simulink model "TosAeb"  (R2021a)
settings: Solver=FixedStepDiscrete  StartTime=0.0  StopTime=inf  FixedStep=0.01  RelTol=1e-3
undefined symbols (not defined in the file or in its folder): ACCModSts, AccSts1, AEB_ObjectMovingOrientation, AEB_ObjectMovingState, CN_BTCActive, CN_CurrentGearR, CN_CurrentGearR_DHT, CN_PTCActive, CN_VDCActive, CN_VSC_EspOff, CN_VSC_FCW_AEB_Error, CN_VSC_FCW_AEB_NoDispay, CN_VSC_FCW_AEB_PerfmDgdtn, CN_VSC_FCW_AEB_TempUnAvlbl, CN_VSC_VehStandstill, CNaccelAvoidMax, CNaccelAvoidMin, CNbrakePedalPress, CNcomfortStrLeftAccelMax, CNcomfortStrLeftAccelMin, CNcomfortStrRightAccelMax, CNcomfortStrRightAccelMin, CNDrvDoorOpen, CNDrvModeEco, CNDrvModeNorm, CNDrvModeOther, CNDrvModeSport, CNDrvSBRreminder, CNNull, CNobjInvalidID, CNPassDoorOpen, CNPassSBRreminder, CNPEBSAccelNegInf, CNPEBSAccelNull, CNPEBSTimeInf, CNPEBSTimeNull, CNSTOPMAX, CNSTOPNULL, CNsubjWidth, CNSwtOFF, CNSwtON, CNTTCMAX, CNTTCNULL, CNVehConfigAEBCycNone, CNVehConfigAEBNone, CNVehConfigAEBPedNone, CNVehConfigFCWNone, CNVehConfigJANone, CollisionSeverity, FALSE, FCWSnvty, JACycObjCalcInfoDefaultParams, JAPedObjCalcInfoDefaultParams, JAVehObjCalcInfoDefaultParams, latCycObjCalcInfoDefaultParams, latPedObjCalcInfoDefaultParams, longObjCalcInfoDefaultParams, ModSts, movingStatus, ObjectType ... (+1112)
libraries: simulink x3031, AEBSFunctionLib x50, Ta_Library x33, AEBSStateMachineLib x23, Mylibrary x12, simulink_extras x7, ObjSelect_lib x3, dspstat3 x2
(part 68 of 102)

== system TosAeb/FcwAebJa_Step_sys/ALG_AEBSystem/ALG_AEBController/AEB_FuncCondCalcApp/CycFunctionCondCalc/cycAEBpartSuppAborCond/cycAEBpartSuppCondCalc/cycAEBpartSituationRelActiveCondCalc/LatDistCond4PartBrakeCalc/LatDistThresCalc/LateralDistanceThresHMISettingLate ==
  vyCorr : Inport
  HMISettingLate : ActionPort  ActionPortLabel=default:
  LateralDistanceThresLookupTable : Lookup_n-D  NumberOfTableDimensions=1  BreakpointsForDimension1=P_AEB_Func_cycAEBpartObjVyAbsLatBoundHmiLateX  BeginIndexSearchUsingPreviousIndexResult=on  Table=P_AEB_Func_cycAEBpartDyLatBoundHmiLateY  InternalRulePriority=Speed  RndMeth=Simplest  InputSameDT=on
  latDistThres : Outport
  wires:
  vyCorr/1 -> LateralDistanceThresLookupTable/1
  LateralDistanceThresLookupTable/1 -> latDistThres/1

== system TosAeb/FcwAebJa_Step_sys/ALG_AEBSystem/ALG_AEBController/AEB_FuncCondCalcApp/LongFunctionCondCalc/vehAEBfullSuppAborCondCalc/ vehAEBfullSuppCondCalc/vehAEBfullVehRelActiveCondCalc/DriverCondJudge/brakeReleventSuppCond/brakeReleventSuppCond_ESP ==
  ESPmasterCylindBrakePress : Inport
  BrakePedalStatus_G : Inport  Port=2
  subjLongAccel : Inport  Port=3
  subjVel : Inport  Port=4
  Action Port : ActionPort  ActionPortLabel=if(u1)
  1-D Lookup Table : Lookup_n-D  NumberOfTableDimensions=1  BreakpointsForDimension1=P_AEB_Func_vehAEBfullBrkAccelActivationThresX  BeginIndexSearchUsingPreviousIndexResult=on  Table=P_AEB_Func_vehAEBfullBrkAccelActivationThresY  ExtrapMethod=Clip  InternalRulePriority=Speed  RndMeth=Simplest  InputSameDT=on
  Compare To Constant2 : simulink/Logic and Bit Operations/Compare To Constant  relop=>  const=P_AEB_Func_vehAEBfullMasterCylindBrkPressActivationThres  OutDataTypeStr=boolean
  Compare To Constant3 : simulink/Logic and Bit Operations/Compare To Constant  relop===  const=CNbrakePedalPress  OutDataTypeStr=boolean
  Logical Operator1 : Logic  Operator=OR  AllPortsSameDT=off  OutDataTypeStr=boolean
  Logical Operator3 : Logic  AllPortsSameDT=off  OutDataTypeStr=boolean
  Relational Operator : RelationalOperator  Operator=<  OutDataTypeStr=boolean  RndMeth=Simplest
  brakeReleventSuppCond : Outport
  wires:
  1-D Lookup Table/1 -> Relational Operator/2
  subjLongAccel/1 -> Relational Operator/1
  BrakePedalStatus_G/1 -> Compare To Constant3/1
  ESPmasterCylindBrakePress/1 -> Compare To Constant2/1
  Compare To Constant2/1 -> Logical Operator1/1
  subjVel/1 -> 1-D Lookup Table/1
  Logical Operator1/1 -> Logical Operator3/1
  Compare To Constant3/1 -> Logical Operator1/2
  Relational Operator/1 -> Logical Operator3/2
  Logical Operator3/1 -> brakeReleventSuppCond/1

== system TosAeb/FcwAebJa_Step_sys/ALG_AEBSystem/ALG_AEBController/AEB_FuncCondCalcApp/LongFunctionCondCalc/vehAEBfullSuppAborCondCalc/ vehAEBfullSuppCondCalc/vehAEBfullVehRelActiveCondCalc/DriverCondJudge/brakeReleventSuppCond/brakeReleventSuppCond_IBooster ==
  note: 0x0:Not applied
      | 0x1:Applied
  Action Port : ActionPort  ActionPortLabel=else
  Constant : Constant  Value=FALSE
  brakeReleventSuppCond : Outport
  wires:
  Constant/1 -> brakeReleventSuppCond/1  "brakeReleventSuppCond"

== system TosAeb/FcwAebJa_Step_sys/ALG_AEBSystem/ALG_AEBController/AEB_FuncCondCalcApp/LongFunctionCondCalc/vehAEBpartSuppAborCondCalc/vehAEBpartSuppCondCalc/vehAEBpartVehRelActiveCondCalc/DriverCondJudge/brakeReleventSuppCond/brakeReleventSuppCond_ESP ==
  ESPmasterCylindBrakePress : Inport
  BrakePedalStatus_G : Inport  Port=2
  Action Port : ActionPort  ActionPortLabel=if(u1)
  Compare To Constant2 : simulink/Logic and Bit Operations/Compare To Constant  relop=>  const=P_AEB_Func_vehAEBpartMasterCylindBrkPressActivationThres  OutDataTypeStr=boolean
  Compare To Constant3 : simulink/Logic and Bit Operations/Compare To Constant  relop===  const=CNbrakePedalPress  OutDataTypeStr=boolean
  Logical Operator1 : Logic  Operator=OR  AllPortsSameDT=off  OutDataTypeStr=boolean
  brakeReleventSuppCond : Outport
  wires:
  Compare To Constant3/1 -> Logical Operator1/2
  Compare To Constant2/1 -> Logical Operator1/1
  ESPmasterCylindBrakePress/1 -> Compare To Constant2/1
  BrakePedalStatus_G/1 -> Compare To Constant3/1
  Logical Operator1/1 -> brakeReleventSuppCond/1  "brakeReleventSuppCond"

== system TosAeb/FcwAebJa_Step_sys/ALG_AEBSystem/ALG_AEBController/AEB_FuncCondCalcApp/LongFunctionCondCalc/vehAEBpartSuppAborCondCalc/vehAEBpartSuppCondCalc/vehAEBpartVehRelActiveCondCalc/DriverCondJudge/brakeReleventSuppCond/brakeReleventSuppCond_IBooster ==
  note: 0x0:Not applied
      | 0x1:Applied
  IB_BrkPedalAppld : Inport
  Action Port : ActionPort  ActionPortLabel=else
  Compare To Constant3 : simulink/Logic and Bit Operations/Compare To Constant  relop===  const=TRUE  OutDataTypeStr=boolean
  brakeReleventSuppCond : Outport
  wires:
  IB_BrkPedalAppld/1 -> Compare To Constant3/1
  Compare To Constant3/1 -> brakeReleventSuppCond/1  "brakeReleventSuppCond"

== system TosAeb/FcwAebJa_Step_sys/ALG_AEBSystem/ALG_AEBController/AEB_FuncCondCalcApp/LongFunctionCondCalc/vehAcuteWarningSuppAborCondFulfilled/vehAcuteWarningSuppCondCalc/vehAcuteWarningVehRelActiveCondCalc/DriverCondJudge/brakeReleventSuppCond/brakeReleventSuppCond_ESP ==
  ESPmasterCylindBrakePress : Inport
  BrakePedalStatus_G : Inport  Port=2
  Action Port : ActionPort  ActionPortLabel=if(u1)
  Compare To Constant2 : simulink/Logic and Bit Operations/Compare To Constant  relop=>  const=P_AEB_Func_vehAcuteWarningMasterCylindBrkPressActivationThres  OutDataTypeStr=boolean
  Compare To Constant3 : simulink/Logic and Bit Operations/Compare To Constant  relop===  const=CNbrakePedalPress  OutDataTypeStr=boolean
  Logical Operator1 : Logic  Operator=OR  AllPortsSameDT=off  OutDataTypeStr=boolean
  brakeReleventSuppCond : Outport
  wires:
  Compare To Constant2/1 -> Logical Operator1/1
  ESPmasterCylindBrakePress/1 -> Compare To Constant2/1
  Logical Operator1/1 -> brakeReleventSuppCond/1  "brakeReleventSuppCond"
  Compare To Constant3/1 -> Logical Operator1/2
  BrakePedalStatus_G/1 -> Compare To Constant3/1

== system TosAeb/FcwAebJa_Step_sys/ALG_AEBSystem/ALG_AEBController/AEB_FuncCondCalcApp/LongFunctionCondCalc/vehAcuteWarningSuppAborCondFulfilled/vehAcuteWarningSuppCondCalc/vehAcuteWarningVehRelActiveCondCalc/DriverCondJudge/brakeReleventSuppCond/brakeReleventSuppCond_IBooster ==
  note: 0x0:Not applied
      | 0x1:Applied
  IB_BrkPedalAppld : Inport
  Action Port : ActionPort  ActionPortLabel=else
  Compare To Constant3 : simulink/Logic and Bit Operations/Compare To Constant  relop===  const=TRUE  OutDataTypeStr=boolean
  brakeReleventSuppCond : Outport
  wires:
  IB_BrkPedalAppld/1 -> Compare To Constant3/1
  Compare To Constant3/1 -> brakeReleventSuppCond/1  "brakeReleventSuppCond"

== system TosAeb/FcwAebJa_Step_sys/ALG_AEBSystem/ALG_AEBController/AEB_FuncCondCalcApp/LongFunctionCondCalc/vehEBASuppAborCondCalc/vehEBASuppCondCalc/vehEBASituationRelActiveCondCalc/brakeReleventSuppCond/brakeReleventSuppCond_ESP/brkTriggerCondCalc1/brkAccelThesLookup ==
  subjVel : Inport  OutDataTypeStr=single
  f : TriggerPort  TriggerType=function-call  VariantControl=Variant
      settings: CopyFcn=Stateflow.SLINSF.SimulinkMan.removeBlockPreDeleteFcn  PreDeleteFcn=Stateflow.SLINSF.SimulinkMan.unsafePredeleteFcn
  BrkAccelThesLookupTable : Lookup_n-D  NumberOfTableDimensions=1  BreakpointsForDimension1=P_AEB_Func_vehEBAObjMovingBrkAccelThresX  Table=P_AEB_Func_vehEBAObjMovingBrkAccelThresY  ExtrapMethod=Clip  InternalRulePriority=Speed  RndMeth=Simplest  InputSameDT=on
  MaxDecel : Outport
  wires:
  BrkAccelThesLookupTable/1 -> MaxDecel/1
  subjVel/1 -> BrkAccelThesLookupTable/1

== system TosAeb/FcwAebJa_Step_sys/ALG_AEBSystem/ALG_AEBController/AEB_FuncCondCalcApp/LongFunctionCondCalc/vehEBASuppAborCondCalc/vehEBASuppCondCalc/vehEBASituationRelActiveCondCalc/brakeReleventSuppCond/brakeReleventSuppCond_IBooster/IB_BrkPedalAppldOpt ==
  IB_BrkPedalAppld : Inport
  Compare To Constant : simulink/Logic and Bit Operations/Compare To Constant  relop=>  const=P_AEB_Func_vehEBAiboosterTime  OutDataTypeStr=boolean
  Constant : Constant  Value=Ts
  Constant1 : Constant  Value=256
  Logical Operator : Logic  AllPortsSameDT=off  OutDataTypeStr=boolean
  timer : AEBSFunctionLib/timer  SystemSampleTime=-1  VariantActivationTime=update diagram  Latency=0  AutoFrameSizeCalculation=off
  IB_BrkPedalAppldOpt : Outport
  wires:
  Compare To Constant/1 -> Logical Operator/2
  Logical Operator/1 -> IB_BrkPedalAppldOpt/1
  timer/1 -> Compare To Constant/1
  Constant/1 -> timer/2
  Constant1/1 -> timer/3
  IB_BrkPedalAppld/1 -> timer/1, Logical Operator/1

== system TosAeb/FcwAebJa_Step_sys/ALG_AEBSystem/ALG_AEBController/AEB_FuncCondCalcApp/LongFunctionCondCalc/vehEBASuppAborCondCalc/vehEBASuppCondCalc/vehEBASituationRelActiveCondCalc/brakeReleventSuppCond/brakeReleventSuppCond_IBooster/brkAccelThesLookup ==
  subjVel : Inport
  BrkAccelThesLookupTable : Lookup_n-D  NumberOfTableDimensions=1  BreakpointsForDimension1=P_Function_vehEBAObjMovingBrkAccelThresX  Table=P_Function_vehEBAObjMovingBrkAccelThresY  ExtrapMethod=Clip  InternalRulePriority=Speed  RndMeth=Simplest  InputSameDT=on
  BrkAccelThes : Outport
  wires:
  BrkAccelThesLookupTable/1 -> BrkAccelThes/1
  subjVel/1 -> BrkAccelThesLookupTable/1

== system TosAeb/FcwAebJa_Step_sys/ALG_AEBSystem/ALG_AEBController/AEB_FuncCondCalcApp/LongFunctionCondCalc/vehEBASuppAborCondCalc/vehEBASuppCondCalc/vehEBASituationRelActiveCondCalc/brakeReleventSuppCond/brakeReleventSuppCond_IBooster/brkTriggerCondCalc1/brkAccelThesLookup ==
  subjVel : Inport  OutDataTypeStr=single
  f : TriggerPort  TriggerType=function-call  VariantControl=Variant
      settings: CopyFcn=Stateflow.SLINSF.SimulinkMan.removeBlockPreDeleteFcn  PreDeleteFcn=Stateflow.SLINSF.SimulinkMan.unsafePredeleteFcn
  BrkAccelThesLookupTable : Lookup_n-D  NumberOfTableDimensions=1  BreakpointsForDimension1=P_AEB_Func_vehEBAObjMovingBrkAccelThresX  Table=P_AEB_Func_vehEBAObjMovingBrkAccelThresY  ExtrapMethod=Clip  InternalRulePriority=Speed  RndMeth=Simplest  InputSameDT=on
  MaxDecel : Outport
  wires:
  subjVel/1 -> BrkAccelThesLookupTable/1
  BrkAccelThesLookupTable/1 -> MaxDecel/1

== system TosAeb/FcwAebJa_Step_sys/ALG_AEBSystem/ALG_AEBController/AEB_FuncCondCalcApp/LongFunctionCondCalc/vehPreWarningSuppAborCondFulfilled/vehPreWarningSuppCondCalc/vehPreWarningVehRelActiveCondCalc/DriverCondJudge/brakeReleventSuppCond/brakeReleventSuppCond_ESP ==
  ESPmasterCylindBrakePress : Inport
  BrakePedalStatus_G : Inport  Port=2
  Action Port : ActionPort  ActionPortLabel=if(u1)
  Compare To Constant2 : simulink/Logic and Bit Operations/Compare To Constant  relop=>  const=P_AEB_Func_vehPreWarningMasterCylindBrkPressThres  OutDataTypeStr=boolean
  Compare To Constant3 : simulink/Logic and Bit Operations/Compare To Constant  relop===  const=CNbrakePedalPress  OutDataTypeStr=boolean
  Logical Operator1 : Logic  Operator=OR  AllPortsSameDT=off  OutDataTypeStr=boolean
  brakeReleventSuppCond : Outport
  wires:
  BrakePedalStatus_G/1 -> Compare To Constant3/1
  Logical Operator1/1 -> brakeReleventSuppCond/1  "brakeReleventSuppCond"
  ESPmasterCylindBrakePress/1 -> Compare To Constant2/1
  Compare To Constant3/1 -> Logical Operator1/2
  Compare To Constant2/1 -> Logical Operator1/1

== system TosAeb/FcwAebJa_Step_sys/ALG_AEBSystem/ALG_AEBController/AEB_FuncCondCalcApp/LongFunctionCondCalc/vehPreWarningSuppAborCondFulfilled/vehPreWarningSuppCondCalc/vehPreWarningVehRelActiveCondCalc/DriverCondJudge/brakeReleventSuppCond/brakeReleventSuppCond_IBooster ==
  note: 0x0:Not applied
      | 0x1:Applied
  IB_BrkPedalAppld : Inport
  Action Port : ActionPort  ActionPortLabel=else
  Compare To Constant3 : simulink/Logic and Bit Operations/Compare To Constant  relop===  const=TRUE  OutDataTypeStr=boolean
  brakeReleventSuppCond : Outport
  wires:
  IB_BrkPedalAppld/1 -> Compare To Constant3/1
  Compare To Constant3/1 -> brakeReleventSuppCond/1  "brakeReleventSuppCond"

== system TosAeb/FcwAebJa_Step_sys/ALG_AEBSystem/ALG_AEBController/AEB_FuncCondCalcApp/LongFunctionCondCalc/vehPrefillSuppAborCondCalc/vehPrefillSuppCondCalc/vehPrefillVehRelActiveCondCalc/DriverCondJudge/brakeReleventSuppCond/brakeReleventSuppCond_ESP ==
  ESPmasterCylindBrakePress : Inport
  BrakePedalStatus_G : Inport  Port=2
  Action Port : ActionPort  ActionPortLabel=if(u1)
  Compare To Constant2 : simulink/Logic and Bit Operations/Compare To Constant  relop=>  const=P_AEB_Func_vehPrefillMasterCylindBrkPressActivationThres  OutDataTypeStr=boolean
  Compare To Constant3 : simulink/Logic and Bit Operations/Compare To Constant  relop===  const=CNbrakePedalPress  OutDataTypeStr=boolean
  Logical Operator1 : Logic  Operator=OR  AllPortsSameDT=off  OutDataTypeStr=boolean
  brakeReleventSuppCond : Outport
  wires:
  Compare To Constant3/1 -> Logical Operator1/2
  BrakePedalStatus_G/1 -> Compare To Constant3/1
  ESPmasterCylindBrakePress/1 -> Compare To Constant2/1
  Logical Operator1/1 -> brakeReleventSuppCond/1  "brakeReleventSuppCond"
  Compare To Constant2/1 -> Logical Operator1/1

== system TosAeb/FcwAebJa_Step_sys/ALG_AEBSystem/ALG_AEBController/AEB_FuncCondCalcApp/LongFunctionCondCalc/vehPrefillSuppAborCondCalc/vehPrefillSuppCondCalc/vehPrefillVehRelActiveCondCalc/DriverCondJudge/brakeReleventSuppCond/brakeReleventSuppCond_IBooster ==
  note: 0x0:Not applied
      | 0x1:Applied
  IB_BrkPedalAppld : Inport
  Action Port : ActionPort  ActionPortLabel=else
  Compare To Constant3 : simulink/Logic and Bit Operations/Compare To Constant  relop===  const=TRUE  OutDataTypeStr=boolean
  brakeReleventSuppCond : Outport
  wires:
  IB_BrkPedalAppld/1 -> Compare To Constant3/1
  Compare To Constant3/1 -> brakeReleventSuppCond/1  "brakeReleventSuppCond"

== system TosAeb/FcwAebJa_Step_sys/ALG_AEBSystem/ALG_AEBController/AEB_FuncImpManagementApp/FuncManeuArbCalc/brkFuncManeuArbCalc/axTgtReqValueCalc1/AebfullTriggerInfoCalc/AEBTriggerRelCalc/AEBTriggerInfo/AEBTriggerInfo/dxSecureCalc ==
  subjVel : Inport
  vxPredicted : Inport  Port=2
  f : TriggerPort  TriggerType=function-call  VariantControl=Variant
      settings: CopyFcn=Stateflow.SLINSF.SimulinkMan.removeBlockPreDeleteFcn  PreDeleteFcn=Stateflow.SLINSF.SimulinkMan.unsafePredeleteFcn
  dxSecureCalc : Subsystem
  dxSecure : Outport
  wires:
  subjVel/1 -> dxSecureCalc/1(subjVel)
  dxSecureCalc/1(dxSecure) -> dxSecure/1
  vxPredicted/1 -> dxSecureCalc/2(vxPredicted)

== system TosAeb/FcwAebJa_Step_sys/ALG_AEBSystem/ALG_AEBController/AEB_FuncImpManagementApp/FuncManeuArbCalc/brkFuncManeuArbCalc/axTgtReqValueCalc1/AebfullTriggerInfoCalc/Subsystem/ObjectPredict/If Action Subsystem ==
  subjVel : Inport
  dxFrontBumper : Inport  Port=2
  vx : Inport  Port=3
  ax : Inport  Port=4
  Action Port : ActionPort  ActionPortLabel=if(u1)
  Bus Creator : BusCreator  Inputs=5
  Bus Creator1 : BusCreator  Inputs=3  OutDataTypeStr=Bus: BusFIM_ObjPredicted  NonVirtualBus=on
      settings: InheritFromInputs=off
  Bus Selector : BusSelector  OutputSignals=tPredicted,dxFrontBumper,vx,ax
  Bus Selector1 : BusSelector  OutputSignals=ax,tPredicted,vx,subjVel
  dxPredictedCalc : Subsystem
  dxPredictedCalc1 : Subsystem
  tPredictedCalc : Subsystem
  ObjectPredicted : Outport
  wires:
  Bus Selector/4 -> dxPredictedCalc/4(ax)  "<ax>"
  dxPredictedCalc1/1(vxAbsObjPredicted) -> Bus Creator1/2  "vxAbsObjPredicted"
  subjVel/1 -> Bus Creator/1  "subjVel"
  Bus Selector/2 -> dxPredictedCalc/2(dxFrontBumper)  "<dxFrontBumper>"
  tPredictedCalc/1(tPredicted) -> Bus Creator/5  "tPredicted"
  Bus Creator/1 -> Bus Selector/1, Bus Selector1/1
  vx/1 -> Bus Creator/3  "vx"
  dxFrontBumper/1 -> Bus Creator/2  "dxFrontBumper"
  Bus Selector1/2 -> dxPredictedCalc1/2(tPredicted)  "<tPredicted>"
  Bus Selector/1 -> dxPredictedCalc/1(tPredicted)  "<tPredicted>"
  ax/1 -> Bus Creator/4  "ax"
  Bus Selector1/3 -> dxPredictedCalc1/3(vx)  "<vx>"
  Bus Creator1/1 -> ObjectPredicted/1
  Bus Selector1/1 -> dxPredictedCalc1/1(ax)  "<ax>"
  Bus Selector/3 -> dxPredictedCalc/3(vx)  "<vx>"
  dxPredictedCalc1/2(vxPredicted) -> Bus Creator1/3  "vxPredicted"
  Bus Selector1/4 -> dxPredictedCalc1/4(subjVel)  "<subjVel>"
  dxPredictedCalc/1(dxFrontBumperPredicted) -> Bus Creator1/1  "dxFrontBumperPredicted"

== system TosAeb/FcwAebJa_Step_sys/ALG_AEBSystem/ALG_AEBController/AEB_FuncImpManagementApp/FuncManeuArbCalc/brkFuncManeuArbCalc/axTgtReqValueCalc1/AebfullTriggerInfoCalc/Subsystem/ObjectPredict/If Action Subsystem1 ==
  vxAbsObj : Inport
  dxFrontBumper : Inport  Port=2
  vx : Inport  Port=3
  Action Port : ActionPort  ActionPortLabel=else
  Bus Creator1 : BusCreator  Inputs=3  OutDataTypeStr=Bus: BusFIM_ObjPredicted  NonVirtualBus=on
      settings: InheritFromInputs=off
  ObjectPredicted : Outport
  wires:
  dxFrontBumper/1 -> Bus Creator1/1  "dxFrontBumperPredicted"
  Bus Creator1/1 -> ObjectPredicted/1
  vx/1 -> Bus Creator1/3  "vxPredicted"
  vxAbsObj/1 -> Bus Creator1/2  "vxAbsObjPredicted"

== system TosAeb/FcwAebJa_Step_sys/ALG_AEBSystem/ALG_AEBController/AEB_FuncImpManagementApp/FuncManeuArbCalc/brkFuncManeuArbCalc/axTgtReqValueCalc1/axTgtReqValueCalc4AebControl/JerkFuncDecCalc/JerkFuncDecCalc/JerkFuncDecCalc/JerkFuncDecCalc ==
  aDesired : Inport
  axTgtReqValueK1 : Inport  Port=2
  accelMin : Inport  Port=3
  JerkFuncDecK1 : Inport  Port=4
  JerkFuncDecMax : Inport  Port=5
  f : TriggerPort  TriggerType=function-call  VariantControl=(inherit)
      settings: CopyFcn=Stateflow.SLINSF.SimulinkMan.removeBlockPreDeleteFcn  PreDeleteFcn=Stateflow.SLINSF.SimulinkMan.unsafePredeleteFcn
  JerkFuncDecCalc : Subsystem
  JerkFuncDecFactor : Subsystem
  aErroraDesiredaTgtReqValue : Subsystem
  JerkFuncDec : Outport
  wires:
  JerkFuncDecMax/1 -> JerkFuncDecCalc/4(JerkFuncDecMax)
  accelMin/1 -> JerkFuncDecFactor/3(accelMin)
  axTgtReqValueK1/1 -> aErroraDesiredaTgtReqValue/1(axTgtReqValueK1)
  JerkFuncDecFactor/1(JerkFuncDecFactor) -> JerkFuncDecCalc/2(JerkFuncDecFactor)
  aErroraDesiredaTgtReqValue/1(aErroraDesiredaTgtReqValue) -> JerkFuncDecCalc/1(aErroraDesiredaTgtReqValue), JerkFuncDecFactor/1(DeltaaDesiredaTgtReqValue)
  aDesired/1 -> aErroraDesiredaTgtReqValue/2(aDesired), JerkFuncDecFactor/2(aDesired)
  JerkFuncDecK1/1 -> JerkFuncDecCalc/3(JerkFuncDecK1)
  JerkFuncDecCalc/1(JerkFuncDec) -> JerkFuncDec/1

== system TosAeb/FcwAebJa_Step_sys/ALG_AEBSystem/ALG_AEBController/AEB_FuncImpManagementApp/FuncManeuArbCalc/brkFuncManeuArbCalc/axTgtReqValueCalc1/axTgtReqValueCalc4AebControl/JerkFuncDecCalc/JerkFuncDecCalc/JerkFuncDecCalc/JerkFuncDecFirstCycleCalc ==
  aDesired : Inport
  JerkFuncDecMax : Inport  Port=2
  f : TriggerPort  TriggerType=function-call  VariantControl=(inherit)
      settings: CopyFcn=Stateflow.SLINSF.SimulinkMan.removeBlockPreDeleteFcn  PreDeleteFcn=Stateflow.SLINSF.SimulinkMan.unsafePredeleteFcn
  1-D Lookup Table : Lookup_n-D  NumberOfTableDimensions=1  BreakpointsForDimension1=P_AEB_FIM_aDesiredJerkInitialX  Table=P_AEB_FIM_aDesiredJerkInitialY  ExtrapMethod=Clip  InternalRulePriority=Speed  RndMeth=Simplest  InputSameDT=on
  Max1 : MinMax  Function=max  Inputs=2
  JerkFuncDecFirstCycle : Outport
  wires:
  aDesired/1 -> 1-D Lookup Table/1
  1-D Lookup Table/1 -> Max1/1  "AEB_FIM_aDesiredJerkInitial"
  Max1/1 -> JerkFuncDecFirstCycle/1
  JerkFuncDecMax/1 -> Max1/2

== system TosAeb/FcwAebJa_Step_sys/ALG_AEBSystem/ALG_AEBController/AEB_FuncImpManagementApp/FuncManeuArbCalc/brkFuncManeuArbCalc/axTgtReqValueCalc1/axTgtReqValueCalc4AebControl/JerkFuncIncCalc/JerkFuncIncCalc/JerkFuncInc4LongBraking ==
  time4IncConstant : Inport
  Action Port : ActionPort  ActionPortLabel=if(u1)
  Constant : Constant  Value=P_AEB_FIM_TimeIncReduceValue4LongBraking
  Divide : Product  Inputs=*/
  JerkFuncInc Lookup Table : Lookup_n-D  NumberOfTableDimensions=1  BreakpointsForDimension1=P_AEB_FIM_Jerk4ControllingIncXTime  Table=P_AEB_FIM_Jerk4ControllingIncY  ExtrapMethod=Clip  InternalRulePriority=Speed  RndMeth=Simplest  InputSameDT=on
  JerkFuncInc : Outport
  wires:
  Divide/1 -> JerkFuncInc Lookup Table/1
  Constant/1 -> Divide/2
  time4IncConstant/1 -> Divide/1
  JerkFuncInc Lookup Table/1 -> JerkFuncInc/1

== system TosAeb/FcwAebJa_Step_sys/ALG_AEBSystem/ALG_AEBController/AEB_FuncImpManagementApp/FuncManeuArbCalc/brkFuncManeuArbCalc/axTgtReqValueCalc1/axTgtReqValueCalc4AebControl/JerkFuncIncCalc/JerkFuncIncCalc/JerkFuncIncAbs ==
  JerkFuncInc : Inport
  Abs : Abs
  JerkFuncIncAbs : Outport
  wires:
  JerkFuncInc/1 -> Abs/1
  Abs/1 -> JerkFuncIncAbs/1

== system TosAeb/FcwAebJa_Step_sys/ALG_AEBSystem/ALG_AEBController/AEB_FuncImpManagementApp/FuncManeuArbCalc/brkFuncManeuArbCalc/axTgtReqValueCalc1/axTgtReqValueCalc4AebControl/JerkFuncIncCalc/JerkFuncIncCalc/JerkFuncIncCalc ==
  time4IncConstant : Inport
  Action Port : ActionPort  ActionPortLabel=else
  JerkFuncInc Lookup Table : Lookup_n-D  NumberOfTableDimensions=1  BreakpointsForDimension1=P_AEB_FIM_Jerk4ControllingIncXTime  Table=P_AEB_FIM_Jerk4ControllingIncY  ExtrapMethod=Clip  InternalRulePriority=Speed  RndMeth=Simplest  InputSameDT=on
  JerkFuncInc : Outport
  wires:
  JerkFuncInc Lookup Table/1 -> JerkFuncInc/1
  time4IncConstant/1 -> JerkFuncInc Lookup Table/1

== system TosAeb/FcwAebJa_Step_sys/ALG_AEBSystem/ALG_AEBController/AEB_FuncImpManagementApp/FuncManeuArbCalc/brkFuncManeuArbCalc/axTgtReqValueCalc1/axTgtReqValueCalc4AebControl/aDesiredCalc/If Action Subsystem1/accelAvoidLowLvlBrkCalc ==
  vx : Inport
  axAbsObj : Inport  Port=2
  tObjStop : Inport  Port=3
  vxAbsObj : Inport  Port=4
  subjVel : Inport  Port=5
  dxEgoBrake : Inport  Port=6
  AccelAvoidSituationJudge : Stateflow chart  TreatAsAtomicUnit=on
  Bus Creator : BusCreator  Inputs=6
  Bus Selector : BusSelector  OutputSignals=vx,axAbsObj
  Bus Selector1 : BusSelector  OutputSignals=dxEgoBrake,vx,tObjStop,subjVel,vxAbsObj,axAbsObj
  Bus Selector2 : BusSelector  OutputSignals=axAbsObj,vx,dxEgoBrake
  Bus Selector3 : BusSelector  OutputSignals=subjVel,vxAbsObj,axAbsObj,dxEgoBrake
  Merge : Merge  Inputs=4
  Situation1 : Subsystem  TreatAsAtomicUnit=on
  Situation2 : Subsystem  TreatAsAtomicUnit=on
  Situation3 : Subsystem  TreatAsAtomicUnit=on
  Situation4 : Subsystem  TreatAsAtomicUnit=on
  accelAvoidLowLvlBrkLimit : Subsystem
  accelAvoidLowLvlBrk : Outport
  wires:
  accelAvoidLowLvlBrkLimit/1(accelAvoidLowLvlBrk) -> accelAvoidLowLvlBrk/1
  Merge/1 -> accelAvoidLowLvlBrkLimit/1(accelAvoidLowLvlBrkNoLimit)
  Bus Selector3/4 -> Situation3/4(dxEgoBrake)  "<dxEgoBrake>"
  Bus Selector3/3 -> Situation3/3(axAbsObj)  "<axAbsObj>"
  Bus Selector3/2 -> Situation3/2(vxAbsObj)  "<vxAbsObj>"
  Bus Selector3/1 -> Situation3/1(subjVel)  "<subjVel>"
  Bus Selector2/1 -> Situation2/1(axAbsObj)  "<axAbsObj>"
  Bus Selector2/2 -> Situation2/2(vx)  "<vx>"
  Bus Selector2/3 -> Situation2/3(dxEgoBrake)  "<dxEgoBrake>"
  Bus Selector/2 -> AccelAvoidSituationJudge/2(axAbsObj)  "<axAbsObj>"
  Bus Selector/1 -> AccelAvoidSituationJudge/1(vx)  "<vx>"
  Bus Creator/1 -> Bus Selector/1, Bus Selector1/1, Bus Selector2/1, Bus Selector3/1
  tObjStop/1 -> Bus Creator/5  "tObjStop"
  axAbsObj/1 -> Bus Creator/2  "axAbsObj"
  vx/1 -> Bus Creator/1  "vx"
  dxEgoBrake/1 -> Bus Creator/6  "dxEgoBrake"
  subjVel/1 -> Bus Creator/4  "subjVel"
  vxAbsObj/1 -> Bus Creator/3  "vxAbsObj"
  [Situation4] -> Situation4/Trigger
  [Situation3] -> Situation3/Trigger
  [Situation2] -> Situation2/Trigger
  AccelAvoidSituationJudge/4(Situation4()) -> [Situation4]
  AccelAvoidSituationJudge/3(Situation3()) -> [Situation3]
  AccelAvoidSituationJudge/2(Situation2()) -> [Situation2]
  Situation4/1(accelAvoidLowLvlBrk) -> Merge/4
  Situation3/1(accelAvoidLowLvlBrk) -> Merge/3
  Situation2/1(accelAvoidLowLvlBrk) -> Merge/2
  Situation1/1(accelAvoidLowLvlBrk) -> Merge/1
  AccelAvoidSituationJudge/1(Situation1()) -> Situation1/Trigger
  Bus Selector1/1 -> Situation1/1(dxEgoBrake)  "<dxEgoBrake>"
  Bus Selector1/2 -> Situation1/2(vx)  "<vx>"
  Bus Selector1/3 -> Situation1/3(tObjStop)  "<tObjStop>"
  Bus Selector1/4 -> Situation1/4(subjVel)  "<subjVel>"
  Bus Selector1/5 -> Situation1/5(vxAbsObj)  "<vxAbsObj>"
  Bus Selector1/6 -> Situation1/6(axAbsObj)  "<axAbsObj>"

== system TosAeb/FcwAebJa_Step_sys/ALG_AEBSystem/ALG_AEBController/AEB_FuncImpManagementApp/FuncManeuArbCalc/brkFuncManeuArbCalc/axTgtReqValueCalc1/axTgtReqValueCalc4AebControl/aDesiredCalc/If Action Subsystem1/dxEgoBrakeCalc ==
  dxFrontBumper : Inport
  dxSecure : Inport  Port=2
  Constant : Constant  Value=CNNull
  Constant1 : Constant  Value=0.01  OutDataTypeStr=single
  MinMax : MinMax  Function=max  Inputs=2
  Relational Operator : RelationalOperator  Operator=<=  OutDataTypeStr=boolean  RndMeth=Simplest
  Subtract : Sum  Inputs=+-
  Switch : Switch  Criteria=u2 ~= 0
  distEgoBrake : Outport
  wires:
  MinMax/1 -> distEgoBrake/1
  Constant/1 -> Switch/1
  dxSecure/1 -> Relational Operator/2, Switch/3
  Subtract/1 -> MinMax/1
  dxFrontBumper/1 -> Subtract/1, Relational Operator/1
  Relational Operator/1 -> Switch/2
  Switch/1 -> Subtract/2
  Constant1/1 -> MinMax/2

== system TosAeb/FcwAebJa_Step_sys/ALG_AEBSystem/ALG_AEBController/AEB_FuncImpManagementApp/FuncManeuArbCalc/brkFuncManeuArbCalc/axTgtReqValueCalc1/axTgtReqValueCalc4AebControl/aDesiredCalc/If Action Subsystem1/tObjStopCalc ==
  accelAbs : Inport
  velAbs : Inport  Port=2
  StopTimeFlow : Stateflow chart  TreatAsAtomicUnit=on
  tStop : Outport
  wires:
  velAbs/1 -> StopTimeFlow/2(velRel)  "velAbs"
  accelAbs/1 -> StopTimeFlow/1(accelRel)  "accelAbs"
  StopTimeFlow/1(tStop) -> tStop/1  "tStop"

== system TosAeb/FcwAebJa_Step_sys/ALG_AEBSystem/ALG_AEBController/AEB_FuncImpManagementApp/FuncManeuArbCalc/brkFuncManeuArbCalc/axTgtReqValueCalc1/axTgtReqValueCalc4AebControl/axTgtReqValueCalc/axTgtReqValueFinal/axTgtReqValueCalc ==
  JerkFuncInc : Inport
  JerkFuncDec : Inport  Port=2
  Ts : Inport  Port=3
  aDesired : Inport  Port=4
  axTgtReqValueK1 : Inport  Port=5
  accelMax : Inport  Port=6
  accelMin : Inport  Port=7
  GradientOpt : Subsystem
  JerkTimeSlot : Subsystem
  axTgtReqValueCalc : Subsystem
  axTgtReqValue : Outport
  wires:
  axTgtReqValueCalc/1(axTgtReqValue) -> axTgtReqValue/1
  accelMin/1 -> axTgtReqValueCalc/5(accelMin)
  accelMax/1 -> axTgtReqValueCalc/4(accelMax)
  JerkTimeSlot/3(aErrorADesiredAxTgtReq) -> axTgtReqValueCalc/3(aErrorADesiredAxTgtReq)
  JerkTimeSlot/2(JerkDecTimeSlot) -> axTgtReqValueCalc/2(JerkDecTimeSlot)
  JerkTimeSlot/1(JerkIncTimeSlot) -> axTgtReqValueCalc/1(JerkIncTimeSlot)
  aDesired/1 -> axTgtReqValueCalc/7(aDesired), JerkTimeSlot/4(aDesired)
  axTgtReqValueK1/1 -> JerkTimeSlot/5(axTgtReqValueK1), axTgtReqValueCalc/6(axTgtReqValueK1)
  Ts/1 -> JerkTimeSlot/3(Ts)
  GradientOpt/2(JerkFuncDecOpt) -> JerkTimeSlot/2(JerkFuncDec)
  GradientOpt/1(JerkFuncIncOpt) -> JerkTimeSlot/1(JerkFuncInc)
  JerkFuncDec/1 -> GradientOpt/2(JerkFuncDec)
  JerkFuncInc/1 -> GradientOpt/1(JerkFuncInc)

== system TosAeb/FcwAebJa_Step_sys/ALG_AEBSystem/ALG_AEBController/AEB_FuncImpManagementApp/FuncManeuArbCalc/brkFuncManeuArbCalc/axTgtReqValueCalc1/axTgtReqValueCalc4AebControl/axTgtReqValueCalc/axTgtReqValueFinal1/axTgtReqValueCalc ==
  JerkFuncInc : Inport
  JerkFuncDec : Inport  Port=2
  Ts : Inport  Port=3
  aDesired : Inport  Port=4
  axTgtReqValueK1 : Inport  Port=5
  accelMax : Inport  Port=6
  accelMin : Inport  Port=7
  GradientOpt : Subsystem
  JerkTimeSlot : Subsystem
  axTgtReqValueCalc : Subsystem
  axTgtReqValue : Outport
  wires:
  axTgtReqValueCalc/1(axTgtReqValue) -> axTgtReqValue/1
  accelMin/1 -> axTgtReqValueCalc/5(accelMin)
  accelMax/1 -> axTgtReqValueCalc/4(accelMax)
  JerkTimeSlot/3(aErrorADesiredAxTgtReq) -> axTgtReqValueCalc/3(aErrorADesiredAxTgtReq)
  JerkTimeSlot/2(JerkDecTimeSlot) -> axTgtReqValueCalc/2(JerkDecTimeSlot)
  JerkTimeSlot/1(JerkIncTimeSlot) -> axTgtReqValueCalc/1(JerkIncTimeSlot)
  aDesired/1 -> axTgtReqValueCalc/7(aDesired), JerkTimeSlot/4(aDesired)
  axTgtReqValueK1/1 -> JerkTimeSlot/5(axTgtReqValueK1), axTgtReqValueCalc/6(axTgtReqValueK1)
  Ts/1 -> JerkTimeSlot/3(Ts)
  GradientOpt/2(JerkFuncDecOpt) -> JerkTimeSlot/2(JerkFuncDec)
  GradientOpt/1(JerkFuncIncOpt) -> JerkTimeSlot/1(JerkFuncInc)
  JerkFuncDec/1 -> GradientOpt/2(JerkFuncDec)
  JerkFuncInc/1 -> GradientOpt/1(JerkFuncInc)

== system TosAeb/FcwAebJa_Step_sys/ALG_AEBSystem/ALG_AEBController/AEB_FuncImpManagementApp/FuncManeuArbCalc/brkFuncManeuArbCalc/axTgtReqValueCalc1/axTgtReqValueCalc4AebControl/axTgtReqValueCalc/velReduceFlgCalc/If Action Subsystem ==
  subjLongAccel : Inport
  Action Port : ActionPort  ActionPortLabel=if(u1)
  Constant : Constant  Value=TRUE
  velReduceFlg : Outport
  axTgtReqValueK1Filt : Outport  Port=2
  wires:
  subjLongAccel/1 -> axTgtReqValueK1Filt/1
  Constant/1 -> velReduceFlg/1

== system TosAeb/FcwAebJa_Step_sys/ALG_AEBSystem/ALG_AEBController/AEB_FuncImpManagementApp/FuncManeuArbCalc/brkFuncManeuArbCalc/axTgtReqValueCalc1/axTgtReqValueCalc4AebControl/axTgtReqValueCalc/velReduceFlgCalc/If Action Subsystem1 ==
  axTgtReqValueK1 : Inport
  Action Port : ActionPort  ActionPortLabel=else
  Constant : Constant  Value=FALSE
  velReduceFlg : Outport
  axTgtReqValueK1Filt : Outport  Port=2
  wires:
  Constant/1 -> velReduceFlg/1
  axTgtReqValueK1/1 -> axTgtReqValueK1Filt/1

== system TosAeb/FcwAebJa_Step_sys/ALG_AEBSystem/ALG_AEBController/AEB_JaObjCalcApp/JAPedTgtInfoCalc/JAPedCollisonAssessInfo/LatPedCollisonAssessInfoCalc/PedDistBrakeFullCalc/DistPhase3Calc/tPhase3Calc/tEgoStopCalc ==
  subjVel : Inport  OutDataTypeStr=single
  subjLongAccelFilt : Inport  Port=2  OutDataTypeStr=single
  tJerkWork : Inport  Port=3  OutDataTypeStr=single
  Kjerk : Inport  Port=4  OutDataTypeStr=single
  accelMax : Inport  Port=5  OutDataTypeStr=single
  Add : Sum  Inputs=3  OutDataTypeStr=single
  Constant : Constant  Value=0.5  OutDataTypeStr=single
  Divide : Product  Inputs=*/  OutDataTypeStr=single
  Gain : Gain  Gain=-1  OutDataTypeStr=single
  Product : Product  Inputs=4  OutDataTypeStr=single
  Product1 : Product  OutDataTypeStr=single
  tEgoStop : Outport
  wires:
  Divide/1 -> Gain/1
  Product/1 -> Add/3
  Product1/1 -> Add/2
  tJerkWork/1 -> Product/2, Product/1, Product1/2
  Add/1 -> Divide/1
  Gain/1 -> tEgoStop/1
  accelMax/1 -> Divide/2
  Kjerk/1 -> Product/3
  subjLongAccelFilt/1 -> Product1/1
  Constant/1 -> Product/4
  subjVel/1 -> Add/1

== system TosAeb/FcwAebJa_Step_sys/ALG_AEBSystem/ALG_AEBController/AEB_JaObjCalcApp/JAPedTgtInfoCalc/JAPedCollisonAssessInfo/LatPedCollisonAssessInfoCalc/PedDistBrakeFullCalc/DistPhase3Calc/tPhase3Calc/tPhase3Calc ==
  tVelDelta : Inport  OutDataTypeStr=single
  tEgoStop : Inport  Port=2  OutDataTypeStr=single
  MinMax : MinMax  Inputs=2  OutDataTypeStr=single
  tPhase3 : Outport
  wires:
  tVelDelta/1 -> MinMax/1
  tEgoStop/1 -> MinMax/2
  MinMax/1 -> tPhase3/1

== system TosAeb/FcwAebJa_Step_sys/ALG_AEBSystem/ALG_AEBController/AEB_JaObjCalcApp/JAPedTgtInfoCalc/JAPedCollisonAssessInfo/LatPedCollisonAssessInfoCalc/PedDistBrakeFullCalc/DistPhase3Calc/tPhase3Calc/tVelDeltaCalc ==
  velDelta : Inport  OutDataTypeStr=single
  subjLongAccelFilt : Inport  Port=2  OutDataTypeStr=single
  tJerkWork : Inport  Port=3  OutDataTypeStr=single
  Kjerk : Inport  Port=4  OutDataTypeStr=single
  accelMax : Inport  Port=5  OutDataTypeStr=single
  Add : Sum  Inputs=+--  OutDataTypeStr=single
  Constant : Constant  Value=0.5  OutDataTypeStr=single
  Divide : Product  Inputs=*/  OutDataTypeStr=single
  Product : Product  Inputs=4  OutDataTypeStr=single
  Product1 : Product  OutDataTypeStr=single
  tVelDelta : Outport
  wires:
  Divide/1 -> tVelDelta/1
  accelMax/1 -> Divide/2
  Kjerk/1 -> Product/3
  velDelta/1 -> Add/1
  Add/1 -> Divide/1
  Product1/1 -> Add/2
  Constant/1 -> Product/4
  tJerkWork/1 -> Product/2, Product/1, Product1/2
  Product/1 -> Add/3
  subjLongAccelFilt/1 -> Product1/1

== system TosAeb/FcwAebJa_Step_sys/ALG_AEBSystem/ALG_AEBController/AEB_JaObjCalcApp/JAPedTgtInfoCalc/JAPedObjCalcInfoCalc/JAPedObjInfoCalc/ObjCrossFlgCal/CollisionProbCalc/Subsystem/ObjBoundingBox ==
  ObjLengthCorr : Inport  OutDataTypeStr=single
  ObjWidthCorr : Inport  Port=2  OutDataTypeStr=single
  dxPreRefPointObj : Inport  Port=3  OutDataTypeStr=single
  dyPreRefPointObj : Inport  Port=4  OutDataTypeStr=single
  sincosCoef : Inport  Port=5
  Bus Creator : BusCreator  Inputs=5
  Mux : Mux
  Mux1 : Mux
  ObjLLCoordinate : Subsystem
  ObjLUCoordinate : Subsystem
  ObjRLCoordinate : Subsystem
  ObjRUCoordinate : Subsystem
  objDxPre : Outport
  objDyPre : Outport  Port=2
  wires:
  ObjLengthCorr/1 -> Bus Creator/1  "ObjLengthCorr"
  ObjWidthCorr/1 -> Bus Creator/2  "ObjWidthCorr"
  dxPreRefPointObj/1 -> Bus Creator/3  "dxPreRefPointObj"
  dyPreRefPointObj/1 -> Bus Creator/4  "dyPreRefPointObj"
  sincosCoef/1 -> Bus Creator/5  "sincosCoef"
  Bus Creator/1 -> ObjRLCoordinate/1(inputSignals), ObjRUCoordinate/1(inputSignals), ObjLLCoordinate/1(inputSignals), ObjLUCoordinate/1(inputSignals)
  ObjLUCoordinate/1(dxPreLUObj) -> [dxPreLUObj]  "dxPreLUObj"
  ObjLUCoordinate/2(dyPreLUobj) -> [dyPreLUObj]  "dyPreLUObj"
  ObjLLCoordinate/1(dxPre_LLObj) -> [dxPreLLObj]  "dxPreLLObj"
  ObjLLCoordinate/2(dyPreLLObj) -> [dyPreLLObj]  "dyPreLLObj"
  ObjRUCoordinate/1(dxPreRUObj) -> [dxPreRUObj]  "dxPreRUObj"
  ObjRUCoordinate/2(dyPreRUObj) -> [dyPreRUObj]  "dyPreRUObj"
  ObjRLCoordinate/1(dxPreRLObj) -> [dxPreRLObj]  "dxPreRLObj"
  ObjRLCoordinate/2(dyPreRLObj) -> [dyPreRLObj]  "dyPreRLObj"
  Mux/1 -> objDxPre/1
  Mux1/1 -> objDyPre/1
  [dxPreRUObj] -> Mux/1  "dxPreRUObj"
  [dxPreLUObj] -> Mux/2
  [dxPreLLObj] -> Mux/3
  [dxPreRLObj] -> Mux/4
  [dyPreRUObj] -> Mux1/1
  [dyPreLUObj] -> Mux1/2
  [dyPreLLObj] -> Mux1/3
  [dyPreRLObj] -> Mux1/4

== system TosAeb/FcwAebJa_Step_sys/ALG_AEBSystem/ALG_AEBController/AEB_JaObjCalcApp/JAPedTgtInfoCalc/JAPedObjCalcInfoCalc/JAPedObjInfoCalc/ObjCrossFlgCal/CollisionProbCalc/Subsystem/ObjLenthWidthCalc ==
  ObjectYawAngle : Inport  OutDataTypeStr=single
  ObjectMovingOrientation : Inport  Port=2
  ObjectLength : Inport  Port=3  OutDataTypeStr=single
  ObjectTypeCameraConfirmed : Inport  Port=4
  ObjectWidth : Inport  Port=5  OutDataTypeStr=single
  ObjLengthWidthCalc : Stateflow chart  TreatAsAtomicUnit=on
  Radians to Degrees : simulink_extras/Transformations/Radians to Degrees
  ObjLengthCorr : Outport
  ObjWidthCorr : Outport  Port=2
  wires:
  ObjectYawAngle/1 -> Radians to Degrees/1
  ObjectLength/1 -> ObjLengthWidthCalc/3(ObjectLength)
  ObjectTypeCameraConfirmed/1 -> ObjLengthWidthCalc/4(ObjectTypeCameraConfirmed)
  ObjectWidth/1 -> ObjLengthWidthCalc/5(ObjectWidth)
  ObjLengthWidthCalc/1(ObjLengthCorr) -> ObjLengthCorr/1
  ObjLengthWidthCalc/2(ObjWidthCorr) -> ObjWidthCorr/1
  ObjectMovingOrientation/1 -> ObjLengthWidthCalc/2(objectMovingOrientation)
  Radians to Degrees/1 -> ObjLengthWidthCalc/1(ObjectYawAngle)

== system TosAeb/FcwAebJa_Step_sys/ALG_AEBSystem/ALG_AEBController/AEB_JaObjCalcApp/JAPedTgtInfoCalc/JAPedObjCalcInfoCalc/JAPedObjInfoCalc/ObjCrossFlgCal/CollisionProbCalc/Subsystem/ObjPreReferPointCalc ==
  dx : Inport  OutDataTypeStr=single
  vxAbsObj : Inport  Port=2  OutDataTypeStr=single
  tPredTime : Inport  Port=3  OutDataTypeStr=single
  vyRelCorr : Inport  Port=4  OutDataTypeStr=single
  dy : Inport  Port=5  OutDataTypeStr=single
  ObjectYawAngleCorr : Inport  Port=6  OutDataTypeStr=single
  Add : Sum  OutDataTypeStr=single
  Add1 : Sum  OutDataTypeStr=single
  Product : Product  OutDataTypeStr=single
  Product2 : Product  OutDataTypeStr=single
  dxPreRefPointObj : Outport
  dyPreRefPointObj : Outport  Port=2
  YawAnglePreObj : Outport  Port=3
  wires:
  vxAbsObj/1 -> Product/1
  tPredTime/1 -> Product2/2, Product/2
  Product/1 -> Add/2
  Add/1 -> dxPreRefPointObj/1
  dx/1 -> Add/1
  vyRelCorr/1 -> Product2/1
  Product2/1 -> Add1/1
  dy/1 -> Add1/2
  Add1/1 -> dyPreRefPointObj/1
  ObjectYawAngleCorr/1 -> YawAnglePreObj/1

== system TosAeb/FcwAebJa_Step_sys/ALG_AEBSystem/ALG_AEBController/AEB_JaObjCalcApp/JAPedTgtInfoCalc/JAPedObjCalcInfoCalc/JAPedObjInfoCalc/ObjCrossFlgCal/CollisionProbCalc/Subsystem/PreCrossFlgCalcFlow/VectorCrossAlgorithm ==
  x1 : Inport  OutDataTypeStr=single
  y1 : Inport  Port=2  OutDataTypeStr=single
  x2 : Inport  Port=3  OutDataTypeStr=single
  y2 : Inport  Port=4  OutDataTypeStr=single
  m1 : Inport  Port=5  OutDataTypeStr=single
  n1 : Inport  Port=6  OutDataTypeStr=single
  m2 : Inport  Port=7  OutDataTypeStr=single
  n2 : Inport  Port=8  OutDataTypeStr=single
  f : TriggerPort  TriggerType=function-call  VariantControl=Variant
      settings: CopyFcn=Stateflow.SLINSF.SimulinkMan.removeBlockPreDeleteFcn  PreDeleteFcn=Stateflow.SLINSF.SimulinkMan.unsafePredeleteFcn
  Abs : Abs
  Bus Creator : BusCreator  Inputs=8
  Bus Selector : BusSelector  OutputSignals=x1,x2,n2,n1,y2,y1,m2,m1
  Compare To Constant : simulink/Logic and Bit Operations/Compare To Constant  relop=<  const=1e-6  OutDataTypeStr=boolean
  If : If  IfExpression=u1
  If Action Subsystem : Subsystem  TreatAsAtomicUnit=on
  If Action Subsystem1 : Subsystem  TreatAsAtomicUnit=on
  Merge : Merge
  VectorMulit : Subsystem
  preCrossFlg : Outport
  wires:
  x1/1 -> Bus Creator/1  "x1"
  y1/1 -> Bus Creator/2  "y1"
  x2/1 -> Bus Creator/3  "x2"
  y2/1 -> Bus Creator/4  "y2"
  m1/1 -> Bus Creator/5  "m1"
  n1/1 -> Bus Creator/6  "n1"
  m2/1 -> Bus Creator/7  "m2"
  n2/1 -> Bus Creator/8  "n2"
  Bus Creator/1 -> If Action Subsystem1/2(Coordinations), Bus Selector/1
  VectorMulit/1(vectorMulit) -> If Action Subsystem1/1(vectorMulit), Abs/1
  Abs/1 -> Compare To Constant/1
  Compare To Constant/1 -> If/1
  If/1 -> If Action Subsystem/Action
  If/2 -> If Action Subsystem1/Action
  If Action Subsystem/1(CrossFlg) -> Merge/1
  If Action Subsystem1/1(CrossFlg) -> Merge/2
  Merge/1 -> preCrossFlg/1
  Bus Selector/1 -> VectorMulit/1(x1)  "<x1>"
  Bus Selector/2 -> VectorMulit/2(x2)  "<x2>"
  Bus Selector/3 -> VectorMulit/3(n2)  "<n2>"
  Bus Selector/4 -> VectorMulit/4(n1)  "<n1>"
  Bus Selector/5 -> VectorMulit/5(y2)  "<y2>"
  Bus Selector/6 -> VectorMulit/6(y1)  "<y1>"
  Bus Selector/7 -> VectorMulit/7(m2)  "<m2>"
  Bus Selector/8 -> VectorMulit/8(m1)  "<m1>"

== system TosAeb/FcwAebJa_Step_sys/ALG_AEBSystem/ALG_AEBController/AEB_JaObjCalcApp/JAPedTgtInfoCalc/JAPedObjCalcInfoCalc/JAPedObjInfoCalc/ObjCrossFlgCal/CollisionProbCalc/Subsystem/PreRefPointEgoCalc ==
  subjVel : Inport  OutDataTypeStr=single
  tPredTime : Inport  Port=2  OutDataTypeStr=single
  subjLongAccel : Inport  Port=3  OutDataTypeStr=single
  subjLatAccel : Inport  Port=4  OutDataTypeStr=single
  subjYawRate : Inport  Port=5  OutDataTypeStr=single
  Add : Sum  OutDataTypeStr=single
  Gain : Gain  Gain=0.5  OutDataTypeStr=single
  Gain1 : Gain  Gain=0.5  OutDataTypeStr=single
  Math Function : Math  Operator=square  SignedPower=on  OutDataTypeStr=single  SaturateOnIntegerOverflow=on
  Product{,1..3} (4 alike) : Product  OutDataTypeStr=single
  " dxPreRefPoint" : Outport
  " dyPreRefPoint" : Outport  Port=2
  YawAnglePre : Outport  Port=3
  wires:
  Gain1/1 -> " dyPreRefPoint"/1
  Gain/1 -> Add/2
  Product3/1 -> YawAnglePre/1
  subjYawRate/1 -> Product3/2
  Product2/1 -> Gain1/1
  subjLatAccel/1 -> Product2/2
  Add/1 -> " dxPreRefPoint"/1
  subjLongAccel/1 -> Product1/2
  Math Function/1 -> Product1/1, Product2/1
  tPredTime/1 -> Product3/1, Math Function/1, Product/2
  subjVel/1 -> Product/1
  Product/1 -> Add/1
  Product1/1 -> Gain/1

== system TosAeb/FcwAebJa_Step_sys/ALG_AEBSystem/ALG_AEBController/AEB_JaObjCalcApp/JAPedTgtInfoCalc/JAPedObjCalcInfoCalc/JAPedObjInfoCalc/ObjCrossFlgCal/CollisionProbCalc/Subsystem/SubjBoundingBox ==
  dxPreRefPoint : Inport  OutDataTypeStr=single
  dyPreRefPoint : Inport  Port=2  OutDataTypeStr=single
  sincosCoef : Inport  Port=3
  Abs : Abs
  Bus Creator : BusCreator  Inputs=6
  Constant : Constant  Value=P_Global_DxvFrontBumper_m  OutDataTypeStr=single
  Constant1 : Constant  Value=P_Global_DxvRearBumper_m  OutDataTypeStr=single
  Constant2 : Constant  Value=P_Global_VehicleWidthMin_m  OutDataTypeStr=single
  Mux : Mux
  Mux1 : Mux
  SubjLLCoordinate : Subsystem
  SubjLUCoordinate : Subsystem
  SubjRLCoordinate : Subsystem
  SubjRUCoordinate : Subsystem
  subjDxPre : Outport
  subjDyPre : Outport  Port=2
  wires:
  Constant2/1 -> Bus Creator/6  "subjWidth"
  Abs/1 -> Bus Creator/5  "dxRearBumperToRearAxis"
  Constant/1 -> Bus Creator/4  "dxFrontBumperToRearAxis"
  sincosCoef/1 -> Bus Creator/3  "sincosCoef"
  dyPreRefPoint/1 -> Bus Creator/2  "dyPreRefPoint"
  dxPreRefPoint/1 -> Bus Creator/1  "dxPreRefPoint"
  Bus Creator/1 -> SubjRLCoordinate/1(inputSignals), SubjRUCoordinate/1(inputSignals), SubjLLCoordinate/1(inputSignals), SubjLUCoordinate/1(inputSignals)
  SubjLUCoordinate/1(dxPreLU) -> [dxPreLU]  "dxPreLU"
  SubjLUCoordinate/2(dyPreLU) -> [dyPreLU]  "dyPreLU"
  SubjLLCoordinate/1(dxPreLL) -> [dxPreLL]  "dxPreLL"
  SubjLLCoordinate/2(dyPreLL) -> [dyPreLL]  "dyPreLL"
  SubjRUCoordinate/1(dxPreRU) -> [dxPreRU]  "dxPreRU"
  SubjRUCoordinate/2(dyPreRU) -> [dyPreRU]  "dyPreRU"
  SubjRLCoordinate/1(dxPreRL) -> [dxPreRL]  "dxPreRL"
  SubjRLCoordinate/2(dyPreRL) -> [dyPreRL]  "dyPreRL"
  Mux/1 -> subjDxPre/1
  Mux1/1 -> subjDyPre/1
  [dxPreRU] -> Mux/1
  [dxPreLU] -> Mux/2
  [dxPreLL] -> Mux/3
  [dxPreRL] -> Mux/4
  [dyPreRU] -> Mux1/1
  [dyPreLU] -> Mux1/2
  [dyPreLL] -> Mux1/3
  [dyPreRL] -> Mux1/4
  Constant1/1 -> Abs/1

== system TosAeb/FcwAebJa_Step_sys/ALG_AEBSystem/ALG_AEBController/AEB_JaObjCalcApp/JAPedTgtInfoCalc/JAPedObjCalcInfoCalc/JAPedObjInfoCalc/ObjCrossFlgCal/CollisionProbCalc/Subsystem/sincosCoefCalc ==
  angle : Inport  OutDataTypeStr=single
  Bus Creator : BusCreator  Inputs=2
  Sincos : Trigonometry  Operator=sincos
  sincosCoef : Outport
  wires:
  Sincos/1 -> Bus Creator/1  "sinCoef"
  angle/1 -> Sincos/1
  Sincos/2 -> Bus Creator/2  "cosCoef"
  Bus Creator/1 -> sincosCoef/1  "sincosCoef"

== system TosAeb/FcwAebJa_Step_sys/ALG_AEBSystem/ALG_AEBController/AEB_JaObjCalcApp/JAPedTgtInfoCalc/JAPedObjCalcInfoCalc/JAPedObjInfoCalc/ObjCrossFlgCal/CollisionProbCalc/Subsystem/sincosCoefCalc1 ==
  angle : Inport  OutDataTypeStr=single
  Bus Creator : BusCreator  Inputs=2
  Sincos : Trigonometry  Operator=sincos
  sincosCoef : Outport
  wires:
  Bus Creator/1 -> sincosCoef/1  "sincosCoef"
  Sincos/2 -> Bus Creator/2  "cosCoef"
  angle/1 -> Sincos/1
  Sincos/1 -> Bus Creator/1  "sinCoef"

== system TosAeb/FcwAebJa_Step_sys/ALG_AEBSystem/ALG_AEBController/AEB_JaObjCalcApp/JAVehTgtInfoCalc/AEBJAVehObjCalc/JAVehObjInfoCalc/ObjCrossFlgCal/CollisionProbCalc/Subsystem/ObjBoundingBoxRear ==
  ObjLengthCorr : Inport  OutDataTypeStr=single
  ObjWidthCorr : Inport  Port=2  OutDataTypeStr=single
  dxPreRefPointObj : Inport  Port=3  OutDataTypeStr=single
  dyPreRefPointObj : Inport  Port=4  OutDataTypeStr=single
  sincosCoef : Inport  Port=5
  Bus Creator : BusCreator  Inputs=5
  Mux : Mux
  Mux1 : Mux
  ObjLLCoordinate : Subsystem
  ObjLUCoordinate : Subsystem
  ObjRLCoordinate : Subsystem
  ObjRUCoordinate : Subsystem
  objDxPre : Outport
  objDyPre : Outport  Port=2
  wires:
  ObjLengthCorr/1 -> Bus Creator/1  "ObjLengthCorr"
  ObjWidthCorr/1 -> Bus Creator/2  "ObjWidthCorr"
  dxPreRefPointObj/1 -> Bus Creator/3  "dxPreRefPointObj"
  dyPreRefPointObj/1 -> Bus Creator/4  "dyPreRefPointObj"
  sincosCoef/1 -> Bus Creator/5  "sincosCoef"
  Bus Creator/1 -> ObjRLCoordinate/1(inputSignals), ObjRUCoordinate/1(inputSignals), ObjLLCoordinate/1(inputSignals), ObjLUCoordinate/1(inputSignals)
  ObjLUCoordinate/1(dxPreLUObj) -> [dxPreLUObj]  "dxPreLUObj"
  ObjLUCoordinate/2(dyPreLUobj) -> [dyPreLUObj]  "dyPreLUObj"
  ObjLLCoordinate/1(dxPre_LLObj) -> [dxPreLLObj]  "dxPreLLObj"
  ObjLLCoordinate/2(dyPreLLObj) -> [dyPreLLObj]  "dyPreLLObj"
  ObjRUCoordinate/1(dxPreRUObj) -> [dxPreRUObj]  "dxPreRUObj"
  ObjRUCoordinate/2(dyPreRUObj) -> [dyPreRUObj]  "dyPreRUObj"
  ObjRLCoordinate/1(dxPreRLObj) -> [dxPreRLObj]  "dxPreRLObj"
  ObjRLCoordinate/2(dyPreRLObj) -> [dyPreRLObj]  "dyPreRLObj"
  Mux/1 -> objDxPre/1
  Mux1/1 -> objDyPre/1
  [dxPreLUObj] -> Mux/2
  [dxPreLLObj] -> Mux/3
  [dxPreRLObj] -> Mux/4
  [dyPreRUObj] -> Mux1/1
  [dyPreLUObj] -> Mux1/2
  [dyPreLLObj] -> Mux1/3
  [dyPreRLObj] -> Mux1/4
  [dxPreRUObj] -> Mux/1

== system TosAeb/FcwAebJa_Step_sys/ALG_AEBSystem/ALG_AEBController/AEB_JaObjCalcApp/JAVehTgtInfoCalc/AEBJAVehObjCalc/JAVehObjInfoCalc/ObjCrossFlgCal/CollisionProbCalc/Subsystem/ObjBoundingBoxfront ==
  ObjLengthCorr : Inport  OutDataTypeStr=single
  ObjWidthCorr : Inport  Port=2  OutDataTypeStr=single
  dxPreRefPointObj : Inport  Port=3  OutDataTypeStr=single
  dyPreRefPointObj : Inport  Port=4  OutDataTypeStr=single
  sincosCoef : Inport  Port=5
  Bus Creator : BusCreator  Inputs=5
  Mux : Mux
  Mux1 : Mux
  ObjLLCoordinate : Subsystem
  ObjLUCoordinate : Subsystem
  ObjRLCoordinate : Subsystem
  ObjRUCoordinate : Subsystem
  objDxPre : Outport
  objDyPre : Outport  Port=2
  wires:
  ObjLengthCorr/1 -> Bus Creator/1  "ObjLengthCorr"
  ObjWidthCorr/1 -> Bus Creator/2  "ObjWidthCorr"
  dxPreRefPointObj/1 -> Bus Creator/3  "dxPreRefPointObj"
  dyPreRefPointObj/1 -> Bus Creator/4  "dyPreRefPointObj"
  sincosCoef/1 -> Bus Creator/5  "sincosCoef"
  Bus Creator/1 -> ObjRLCoordinate/1(inputSignals), ObjRUCoordinate/1(inputSignals), ObjLLCoordinate/1(inputSignals), ObjLUCoordinate/1(inputSignals)
  ObjLUCoordinate/1(dxPreLUObj) -> [dxPreLUObj]  "dxPreLUObj"
  ObjLUCoordinate/2(dyPreLUobj) -> [dyPreLUObj]  "dyPreLUObj"
  ObjLLCoordinate/1(dxPre_LLObj) -> [dxPreLLObj]  "dxPreLLObj"
  ObjLLCoordinate/2(dyPreLLObj) -> [dyPreLLObj]  "dyPreLLObj"
  ObjRUCoordinate/1(dxPreRUObj) -> [dxPreRUObj]  "dxPreRUObj"
  ObjRUCoordinate/2(dyPreRUObj) -> [dyPreRUObj]  "dyPreRUObj"
  ObjRLCoordinate/1(dxPreRLObj) -> [dxPreRLObj]  "dxPreRLObj"
  ObjRLCoordinate/2(dyPreRLObj) -> [dyPreRLObj]  "dyPreRLObj"
  Mux/1 -> objDxPre/1
  Mux1/1 -> objDyPre/1
  [dxPreLUObj] -> Mux/2
  [dxPreLLObj] -> Mux/3
  [dxPreRLObj] -> Mux/4
  [dyPreRUObj] -> Mux1/1
  [dyPreLUObj] -> Mux1/2
  [dyPreLLObj] -> Mux1/3
  [dyPreRLObj] -> Mux1/4
  [dxPreRUObj] -> Mux/1

== system TosAeb/FcwAebJa_Step_sys/ALG_AEBSystem/ALG_AEBController/AEB_JaObjCalcApp/JAVehTgtInfoCalc/AEBJAVehObjCalc/JAVehObjInfoCalc/ObjCrossFlgCal/CollisionProbCalc/Subsystem/ObjLenthWidthCalc ==
  ObjectYawAngle : Inport  OutDataTypeStr=single
  ObjectMovingOrientation : Inport  Port=2
  ObjectLength : Inport  Port=3  OutDataTypeStr=single
  FusionType : Inport  Port=4
  ObjectWidth : Inport  Port=5  OutDataTypeStr=single
  ObjLengthWidthCalc : Stateflow chart  TreatAsAtomicUnit=on
  P_JaVehCA_PrecollisionVehLengthThres : Constant  Value=P_JaVehCA_PrecollisionVehLengthThres
  P_JaVehCA_PrecollisionVehWidthThres : Constant  Value=P_JaVehCA_PrecollisionVehWidthThres
  ObjLengthCorr : Outport
  ObjWidthCorr : Outport  Port=2
  wires:
  ObjectYawAngle/1 -> ObjLengthWidthCalc/1(ObjectYawAngle)
  ObjectLength/1 -> ObjLengthWidthCalc/3(ObjectLength)
  FusionType/1 -> ObjLengthWidthCalc/4(FusionType)
  ObjectWidth/1 -> ObjLengthWidthCalc/5(ObjectWidth)
  ObjLengthWidthCalc/1(ObjLengthCorr) -> ObjLengthCorr/1
  ObjLengthWidthCalc/2(ObjWidthCorr) -> ObjWidthCorr/1
  ObjectMovingOrientation/1 -> ObjLengthWidthCalc/2(objectMovingOrientation)
  P_JaVehCA_PrecollisionVehLengthThres/1 -> ObjLengthWidthCalc/6(P_JaVehCA_PrecollisionVehLengthThres)
  P_JaVehCA_PrecollisionVehWidthThres/1 -> ObjLengthWidthCalc/7(P_JaVehCA_PrecollisionVehWidthThres)
